simulink model "wlan_phy_rx_pmd"  (Simulink 7.8)
settings: Solver=VariableStepDiscrete  SolverMode=SingleTasking  StartTime=0.0  StopTime=rx_sim.sim_time  RelTol=1e-3
PostLoadFcn:
  | wlan_phy_rx_init
InitFcn:
  | wlan_phy_rx_init
StartFcn:
  | wlan_phy_rx_init
values from neighbouring files (symbols this model uses):
  barker_seq20 = 0.95 * circshift(barker_seq20, [0 4]) ./ max(abs(barker_seq20))    [wlan_phy_rx_init.m]
  bit_scrambler_lfsr_bytes = sum(bit_scrambler_lfsr_bytes .* repmat(2.^[0:7], 127, 1),2)    [wlan_phy_rx_init.m]
  longCorr_coef_i = [3*real(longCorr_coef)]    [wlan_phy_rx_init.m]
  longCorr_coef_q = [3*imag(longCorr_coef)]    [wlan_phy_rx_init.m]
  MAX_CP_LEN = 32    [wlan_phy_rx_init.m]
  MAX_NCBPS = NCBPS_11n_64QAM    (= 312)    [wlan_phy_rx_init.m]
  MAX_NUM_BYTES = 2^16-1    (= 65535)    [wlan_phy_rx_init.m]
  MAX_NUM_SC = 64    [wlan_phy_rx_init.m]
  MAX_NUM_SYMS = 600    [wlan_phy_rx_init.m]
  NDBPS_11a_BPSK12 = 24    [wlan_phy_rx_init.m]
  preFFT_sampBuff_numSamps = 4*MAX_NUM_SC    (= 256)    [wlan_phy_rx_init.m]
  rx_sim = struct()    [wlan_phy_rx_init.m]
  scr_ind_rev = zeros(1,128)    [wlan_phy_rx_init.m]
undefined symbols (not defined in the file or in its folder): gcbh, mcs_rom_11ag, mcs_rom_11n, wlan_rx_deinterleave_rom_gen, xlBlockLoadCallback, xlGetNormalizedPeriod, xlipgetpartsetting
libraries: xbsIndex_r4 x4943, xbsEDKLib_r4 x1
(part 17 of 28)

== system wlan_phy_rx_pmd/Sync & Antenna Sel/LTS Correlation/Correlator/C5 ==
  x : Inport
  x_valid : Inport  Port=2
  AddSub{1,4..7,12..13} (7 alike) : xbsIndex_r4/AddSub  mode=Addition  use_carryin=off  use_carryout=off  en=off  latency=1  precision=Full  arith_type=Unsigned  n_bits=8  bin_pt=2  quantization=Truncate  overflow=Wrap  dbl_ovrd=off  use_behavioral_HDL=on  hw_selection=Fabric  pipelined=off  use_rpm=off  block_type=addsub
      AddSub1 settings: xl_area=[3 6 0 5 0 0 0]  block_version=VER_STRING_GOES_HERE
      AddSub12 settings: xl_area=[3 5 0 4 0 0 0]  block_version=VER_STRING_GOES_HERE
      AddSub13 settings: xl_area=[3 6 0 5 0 0 0]  block_version=VER_STRING_GOES_HERE
      AddSub4 settings: xl_area=[4 7 0 6 0 0 0]  block_version=VER_STRING_GOES_HERE
      AddSub5 settings: xl_area=[3 6 0 5 0 0 0]  block_version=VER_STRING_GOES_HERE
      AddSub6 settings: xl_area=[3 5 0 4 0 0 0]  block_version=VER_STRING_GOES_HERE
      AddSub7 settings: xl_area=[3 5 0 4 0 0 0]  block_version=VER_STRING_GOES_HERE
  C1 : Subsystem  mask{h=h, c_ind=0, N=N}
      mask prompts: h "Coefficients (all)"; c_ind "Sub-correlator Ind"; N "Coef/correlator"
      mask init:
      | coef2 = h((c_ind*N+1) : (c_ind + 1)*N);
  C2 : Subsystem  mask{h=h, c_ind=1, N=N}
      mask prompts: h "Coefficients (all)"; c_ind "Sub-correlator Ind"; N "Coef/correlator"
      mask init:
      | coef2 = h((c_ind*N+1) : (c_ind + 1)*N);
  C3 : Subsystem  mask{h=h, c_ind=2, N=N}
      mask prompts: h "Coefficients (all)"; c_ind "Sub-correlator Ind"; N "Coef/correlator"
      mask init:
      | coef2 = h((c_ind*N+1) : (c_ind + 1)*N);
  C4 : Subsystem  mask{h=h, c_ind=3, N=N}
      mask prompts: h "Coefficients (all)"; c_ind "Sub-correlator Ind"; N "Coef/correlator"
      mask init:
      | coef2 = h((c_ind*N+1) : (c_ind + 1)*N);
  C5 : Subsystem  mask{h=h, c_ind=4, N=N}
      mask prompts: h "Coefficients (all)"; c_ind "Sub-correlator Ind"; N "Coef/correlator"
      mask init:
      | coef2 = h((c_ind*N+1) : (c_ind + 1)*N);
  C6 : Subsystem  mask{h=h, c_ind=5, N=N}
      mask prompts: h "Coefficients (all)"; c_ind "Sub-correlator Ind"; N "Coef/correlator"
      mask init:
      | coef2 = h((c_ind*N+1) : (c_ind + 1)*N);
  C7 : Subsystem  mask{h=h, c_ind=6, N=N}
      mask prompts: h "Coefficients (all)"; c_ind "Sub-correlator Ind"; N "Coef/correlator"
      mask init:
      | coef2 = h((c_ind*N+1) : (c_ind + 1)*N);
  C8 : Subsystem  mask{h=h, c_ind=7, N=N}
      mask prompts: h "Coefficients (all)"; c_ind "Sub-correlator Ind"; N "Coef/correlator"
      mask init:
      | coef2 = h((c_ind*N+1) : (c_ind + 1)*N);
  Gateway Out{1..14} (14 alike) : xbsIndex_r4/Gateway Out  inherit_from_input=off  hdl_port=off  timing_constraint=None  locs_specified=off  LOCs={}  UseAsDAC=off  DACChannel='1'  block_type=gatewayout
      settings: infoedit="Gateway out block.  Converts Xilinx fixed point inputs into ouputs of type Simulink integer, double, or fixed point.<P><P>Hardware notes:  In hardware these blocks become top level output ports or are discarded, depending on how they are configured."  xl_area=[0 0 0 0 0 0 0]  block_version=VER_STRING_GOES_HERE
  y : Outport
  y_vout : Outport  Port=2
  x_dly : Outport  Port=3
  x_dly_vout : Outport  Port=4
  wires:
  C8/2(x_dly_vout) -> x_dly_vout/1
  C8/1(x_dly) -> x_dly/1
  Gateway Out14/1 -> ?
  Gateway Out13/1 -> ?
  Gateway Out12/1 -> ?
  Gateway Out11/1 -> ?
  Gateway Out10/1 -> ?
  Gateway Out9/1 -> ?
  Gateway Out1/1 -> ?
  Gateway Out8/1 -> ?
  Gateway Out6/1 -> ?
  Gateway Out7/1 -> ?
  C8/4(y_vout) -> y_vout/1
  AddSub4/1 -> Gateway Out14/1, y/1, Gateway Out5/1
  AddSub1/1 -> AddSub4/1
  AddSub13/1 -> AddSub4/2
  AddSub7/1 -> AddSub1/2
  AddSub6/1 -> AddSub1/1
  C7/3(y) -> Gateway Out12/1, AddSub7/1
  C8/3(y) -> Gateway Out13/1, AddSub7/2
  C5/3(y) -> Gateway Out10/1, AddSub6/1
  C6/3(y) -> Gateway Out11/1, AddSub6/2
  C7/2(x_dly_vout) -> C8/2(vin)
  C7/1(x_dly) -> C8/1(x)
  C6/2(x_dly_vout) -> C7/2(vin)
  C6/1(x_dly) -> C7/1(x)
  C5/2(x_dly_vout) -> C6/2(vin)
  C5/1(x_dly) -> C6/1(x)
  C4/3(y) -> Gateway Out9/1, AddSub12/2
  C4/2(x_dly_vout) -> C5/2(vin)
  C4/1(x_dly) -> C5/1(x)
  C3/3(y) -> Gateway Out8/1, AddSub12/1
  C3/2(x_dly_vout) -> C4/2(vin)
  C3/1(x_dly) -> C4/1(x)
  C1/3(y) -> Gateway Out7/1, Gateway Out2/1, AddSub5/1
  C2/3(y) -> Gateway Out1/1, AddSub5/2
  C2/2(x_dly_vout) -> C3/2(vin)
  C2/1(x_dly) -> C3/1(x)
  C1/2(x_dly_vout) -> C2/2(vin)
  C1/1(x_dly) -> C2/1(x)
  x_valid/1 -> Gateway Out4/1, C1/2(vin)
  ? -> ?
  Gateway Out2/1 -> ?
  Gateway Out5/1 -> ?
  Gateway Out3/1 -> ?
  Gateway Out4/1 -> ?
  AddSub5/1 -> AddSub13/1
  AddSub12/1 -> AddSub13/2
  x/1 -> Gateway Out6/1, Gateway Out3/1, C1/1(x)

== system wlan_phy_rx_pmd/Sync & Antenna Sel/LTS Correlation/Correlator/C6 ==
  x : Inport
  x_valid : Inport  Port=2
  AddSub{1,4..7,12..13} (7 alike) : xbsIndex_r4/AddSub  mode=Addition  use_carryin=off  use_carryout=off  en=off  latency=1  precision=Full  arith_type=Unsigned  n_bits=8  bin_pt=2  quantization=Truncate  overflow=Wrap  dbl_ovrd=off  use_behavioral_HDL=on  hw_selection=Fabric  pipelined=off  use_rpm=off  block_type=addsub
      AddSub1 settings: xl_area=[3 6 0 5 0 0 0]  block_version=VER_STRING_GOES_HERE
      AddSub12 settings: xl_area=[3 5 0 4 0 0 0]  block_version=VER_STRING_GOES_HERE
      AddSub13 settings: xl_area=[3 6 0 5 0 0 0]  block_version=VER_STRING_GOES_HERE
      AddSub4 settings: xl_area=[4 7 0 6 0 0 0]  block_version=VER_STRING_GOES_HERE
      AddSub5 settings: xl_area=[3 6 0 5 0 0 0]  block_version=VER_STRING_GOES_HERE
      AddSub6 settings: xl_area=[3 5 0 4 0 0 0]  block_version=VER_STRING_GOES_HERE
      AddSub7 settings: xl_area=[3 5 0 4 0 0 0]  block_version=VER_STRING_GOES_HERE
  C1 : Subsystem  mask{h=h, c_ind=0, N=N}
      mask prompts: h "Coefficients (all)"; c_ind "Sub-correlator Ind"; N "Coef/correlator"
      mask init:
      | coef2 = h((c_ind*N+1) : (c_ind + 1)*N);
  C2 : Subsystem  mask{h=h, c_ind=1, N=N}
      mask prompts: h "Coefficients (all)"; c_ind "Sub-correlator Ind"; N "Coef/correlator"
      mask init:
      | coef2 = h((c_ind*N+1) : (c_ind + 1)*N);
  C3 : Subsystem  mask{h=h, c_ind=2, N=N}
      mask prompts: h "Coefficients (all)"; c_ind "Sub-correlator Ind"; N "Coef/correlator"
      mask init:
      | coef2 = h((c_ind*N+1) : (c_ind + 1)*N);
  C4 : Subsystem  mask{h=h, c_ind=3, N=N}
      mask prompts: h "Coefficients (all)"; c_ind "Sub-correlator Ind"; N "Coef/correlator"
      mask init:
      | coef2 = h((c_ind*N+1) : (c_ind + 1)*N);
  C5 : Subsystem  mask{h=h, c_ind=4, N=N}
      mask prompts: h "Coefficients (all)"; c_ind "Sub-correlator Ind"; N "Coef/correlator"
      mask init:
      | coef2 = h((c_ind*N+1) : (c_ind + 1)*N);
  C6 : Subsystem  mask{h=h, c_ind=5, N=N}
      mask prompts: h "Coefficients (all)"; c_ind "Sub-correlator Ind"; N "Coef/correlator"
      mask init:
      | coef2 = h((c_ind*N+1) : (c_ind + 1)*N);
  C7 : Subsystem  mask{h=h, c_ind=6, N=N}
      mask prompts: h "Coefficients (all)"; c_ind "Sub-correlator Ind"; N "Coef/correlator"
      mask init:
      | coef2 = h((c_ind*N+1) : (c_ind + 1)*N);
  C8 : Subsystem  mask{h=h, c_ind=7, N=N}
      mask prompts: h "Coefficients (all)"; c_ind "Sub-correlator Ind"; N "Coef/correlator"
      mask init:
      | coef2 = h((c_ind*N+1) : (c_ind + 1)*N);
  Gateway Out{1..14} (14 alike) : xbsIndex_r4/Gateway Out  inherit_from_input=off  hdl_port=off  timing_constraint=None  locs_specified=off  LOCs={}  UseAsDAC=off  DACChannel='1'  block_type=gatewayout
      settings: infoedit="Gateway out block.  Converts Xilinx fixed point inputs into ouputs of type Simulink integer, double, or fixed point.<P><P>Hardware notes:  In hardware these blocks become top level output ports or are discarded, depending on how they are configured."  xl_area=[0 0 0 0 0 0 0]  block_version=VER_STRING_GOES_HERE
  y : Outport
  y_vout : Outport  Port=2
  x_dly : Outport  Port=3
  x_dly_vout : Outport  Port=4
  wires:
  x/1 -> C1/1(x), Gateway Out3/1, Gateway Out6/1
  AddSub12/1 -> AddSub13/2
  AddSub5/1 -> AddSub13/1
  Gateway Out4/1 -> ?
  Gateway Out3/1 -> ?
  Gateway Out5/1 -> ?
  Gateway Out2/1 -> ?
  ? -> ?
  x_valid/1 -> C1/2(vin), Gateway Out4/1
  C1/1(x_dly) -> C2/1(x)
  C1/2(x_dly_vout) -> C2/2(vin)
  C2/1(x_dly) -> C3/1(x)
  C2/2(x_dly_vout) -> C3/2(vin)
  C2/3(y) -> AddSub5/2, Gateway Out1/1
  C1/3(y) -> AddSub5/1, Gateway Out2/1, Gateway Out7/1
  C3/1(x_dly) -> C4/1(x)
  C3/2(x_dly_vout) -> C4/2(vin)
  C3/3(y) -> AddSub12/1, Gateway Out8/1
  C4/1(x_dly) -> C5/1(x)
  C4/2(x_dly_vout) -> C5/2(vin)
  C4/3(y) -> AddSub12/2, Gateway Out9/1
  C5/1(x_dly) -> C6/1(x)
  C5/2(x_dly_vout) -> C6/2(vin)
  C6/1(x_dly) -> C7/1(x)
  C6/2(x_dly_vout) -> C7/2(vin)
  C7/1(x_dly) -> C8/1(x)
  C7/2(x_dly_vout) -> C8/2(vin)
  C6/3(y) -> AddSub6/2, Gateway Out11/1
  C5/3(y) -> AddSub6/1, Gateway Out10/1
  C8/3(y) -> AddSub7/2, Gateway Out13/1
  C7/3(y) -> AddSub7/1, Gateway Out12/1
  AddSub6/1 -> AddSub1/1
  AddSub7/1 -> AddSub1/2
  AddSub13/1 -> AddSub4/2
  AddSub1/1 -> AddSub4/1
  AddSub4/1 -> Gateway Out5/1, y/1, Gateway Out14/1
  C8/4(y_vout) -> y_vout/1
  Gateway Out7/1 -> ?
  Gateway Out6/1 -> ?
  Gateway Out8/1 -> ?
  Gateway Out1/1 -> ?
  Gateway Out9/1 -> ?
  Gateway Out10/1 -> ?
  Gateway Out11/1 -> ?
  Gateway Out12/1 -> ?
  Gateway Out13/1 -> ?
  Gateway Out14/1 -> ?
  C8/1(x_dly) -> x_dly/1
  C8/2(x_dly_vout) -> x_dly_vout/1

== system wlan_phy_rx_pmd/Sync & Antenna Sel/LTS Correlation/Correlator/C7 ==
  x : Inport
  x_valid : Inport  Port=2
  AddSub{1,4..7,12..13} (7 alike) : xbsIndex_r4/AddSub  mode=Addition  use_carryin=off  use_carryout=off  en=off  latency=1  precision=Full  arith_type=Unsigned  n_bits=8  bin_pt=2  quantization=Truncate  overflow=Wrap  dbl_ovrd=off  use_behavioral_HDL=on  hw_selection=Fabric  pipelined=off  use_rpm=off  block_type=addsub
      AddSub1 settings: xl_area=[3 6 0 5 0 0 0]  block_version=VER_STRING_GOES_HERE
      AddSub12 settings: xl_area=[3 5 0 4 0 0 0]  block_version=VER_STRING_GOES_HERE
      AddSub13 settings: xl_area=[3 6 0 5 0 0 0]  block_version=VER_STRING_GOES_HERE
      AddSub4 settings: xl_area=[4 7 0 6 0 0 0]  block_version=VER_STRING_GOES_HERE
      AddSub5 settings: xl_area=[3 6 0 5 0 0 0]  block_version=VER_STRING_GOES_HERE
      AddSub6 settings: xl_area=[3 5 0 4 0 0 0]  block_version=VER_STRING_GOES_HERE
      AddSub7 settings: xl_area=[3 5 0 4 0 0 0]  block_version=VER_STRING_GOES_HERE
  C1 : Subsystem  mask{h=h, c_ind=0, N=N}
      mask prompts: h "Coefficients (all)"; c_ind "Sub-correlator Ind"; N "Coef/correlator"
      mask init:
      | coef2 = h((c_ind*N+1) : (c_ind + 1)*N);
  C2 : Subsystem  mask{h=h, c_ind=1, N=N}
      mask prompts: h "Coefficients (all)"; c_ind "Sub-correlator Ind"; N "Coef/correlator"
      mask init:
      | coef2 = h((c_ind*N+1) : (c_ind + 1)*N);
  C3 : Subsystem  mask{h=h, c_ind=2, N=N}
      mask prompts: h "Coefficients (all)"; c_ind "Sub-correlator Ind"; N "Coef/correlator"
      mask init:
      | coef2 = h((c_ind*N+1) : (c_ind + 1)*N);
  C4 : Subsystem  mask{h=h, c_ind=3, N=N}
      mask prompts: h "Coefficients (all)"; c_ind "Sub-correlator Ind"; N "Coef/correlator"
      mask init:
      | coef2 = h((c_ind*N+1) : (c_ind + 1)*N);
  C5 : Subsystem  mask{h=h, c_ind=4, N=N}
      mask prompts: h "Coefficients (all)"; c_ind "Sub-correlator Ind"; N "Coef/correlator"
      mask init:
      | coef2 = h((c_ind*N+1) : (c_ind + 1)*N);
  C6 : Subsystem  mask{h=h, c_ind=5, N=N}
      mask prompts: h "Coefficients (all)"; c_ind "Sub-correlator Ind"; N "Coef/correlator"
      mask init:
      | coef2 = h((c_ind*N+1) : (c_ind + 1)*N);
  C7 : Subsystem  mask{h=h, c_ind=6, N=N}
      mask prompts: h "Coefficients (all)"; c_ind "Sub-correlator Ind"; N "Coef/correlator"
      mask init:
      | coef2 = h((c_ind*N+1) : (c_ind + 1)*N);
  C8 : Subsystem  mask{h=h, c_ind=7, N=N}
      mask prompts: h "Coefficients (all)"; c_ind "Sub-correlator Ind"; N "Coef/correlator"
      mask init:
      | coef2 = h((c_ind*N+1) : (c_ind + 1)*N);
  Gateway Out{1..14} (14 alike) : xbsIndex_r4/Gateway Out  inherit_from_input=off  hdl_port=off  timing_constraint=None  locs_specified=off  LOCs={}  UseAsDAC=off  DACChannel='1'  block_type=gatewayout
      settings: infoedit="Gateway out block.  Converts Xilinx fixed point inputs into ouputs of type Simulink integer, double, or fixed point.<P><P>Hardware notes:  In hardware these blocks become top level output ports or are discarded, depending on how they are configured."  xl_area=[0 0 0 0 0 0 0]  block_version=VER_STRING_GOES_HERE
  y : Outport
  y_vout : Outport  Port=2
  x_dly : Outport  Port=3
  x_dly_vout : Outport  Port=4
  wires:
  x/1 -> C1/1(x), Gateway Out3/1, Gateway Out6/1
  AddSub12/1 -> AddSub13/2
  AddSub5/1 -> AddSub13/1
  Gateway Out4/1 -> ?
  Gateway Out3/1 -> ?
  Gateway Out5/1 -> ?
  Gateway Out2/1 -> ?
  ? -> ?
  x_valid/1 -> C1/2(vin), Gateway Out4/1
  C1/1(x_dly) -> C2/1(x)
  C1/2(x_dly_vout) -> C2/2(vin)
  C2/1(x_dly) -> C3/1(x)
  C2/2(x_dly_vout) -> C3/2(vin)
  C2/3(y) -> AddSub5/2, Gateway Out1/1
  C1/3(y) -> AddSub5/1, Gateway Out2/1, Gateway Out7/1
  C3/1(x_dly) -> C4/1(x)
  C3/2(x_dly_vout) -> C4/2(vin)
  C3/3(y) -> AddSub12/1, Gateway Out8/1
  C4/1(x_dly) -> C5/1(x)
  C4/2(x_dly_vout) -> C5/2(vin)
  C4/3(y) -> AddSub12/2, Gateway Out9/1
  C5/1(x_dly) -> C6/1(x)
  C5/2(x_dly_vout) -> C6/2(vin)
  C6/1(x_dly) -> C7/1(x)
  C6/2(x_dly_vout) -> C7/2(vin)
  C7/1(x_dly) -> C8/1(x)
  C7/2(x_dly_vout) -> C8/2(vin)
  C6/3(y) -> AddSub6/2, Gateway Out11/1
  C5/3(y) -> AddSub6/1, Gateway Out10/1
  C8/3(y) -> AddSub7/2, Gateway Out13/1
  C7/3(y) -> AddSub7/1, Gateway Out12/1
  AddSub6/1 -> AddSub1/1
  AddSub7/1 -> AddSub1/2
  AddSub13/1 -> AddSub4/2
  AddSub1/1 -> AddSub4/1
  AddSub4/1 -> Gateway Out5/1, y/1, Gateway Out14/1
  C8/4(y_vout) -> y_vout/1
  Gateway Out7/1 -> ?
  Gateway Out6/1 -> ?
  Gateway Out8/1 -> ?
  Gateway Out1/1 -> ?
  Gateway Out9/1 -> ?
  Gateway Out10/1 -> ?
  Gateway Out11/1 -> ?
  Gateway Out12/1 -> ?
  Gateway Out13/1 -> ?
  Gateway Out14/1 -> ?
  C8/1(x_dly) -> x_dly/1
  C8/2(x_dly_vout) -> x_dly_vout/1

== system wlan_phy_rx_pmd/Sync & Antenna Sel/LTS Correlation/Correlator/C8 ==
  x : Inport
  x_valid : Inport  Port=2
  AddSub{1,4..7,12..13} (7 alike) : xbsIndex_r4/AddSub  mode=Addition  use_carryin=off  use_carryout=off  en=off  latency=1  precision=Full  arith_type=Unsigned  n_bits=8  bin_pt=2  quantization=Truncate  overflow=Wrap  dbl_ovrd=off  use_behavioral_HDL=on  hw_selection=Fabric  pipelined=off  use_rpm=off  block_type=addsub
      AddSub1 settings: xl_area=[3 6 0 5 0 0 0]  block_version=VER_STRING_GOES_HERE
      AddSub12 settings: xl_area=[3 5 0 4 0 0 0]  block_version=VER_STRING_GOES_HERE
      AddSub13 settings: xl_area=[3 6 0 5 0 0 0]  block_version=VER_STRING_GOES_HERE
      AddSub4 settings: xl_area=[4 7 0 6 0 0 0]  block_version=VER_STRING_GOES_HERE
      AddSub5 settings: xl_area=[3 6 0 5 0 0 0]  block_version=VER_STRING_GOES_HERE
      AddSub6 settings: xl_area=[3 5 0 4 0 0 0]  block_version=VER_STRING_GOES_HERE
      AddSub7 settings: xl_area=[3 5 0 4 0 0 0]  block_version=VER_STRING_GOES_HERE
  C1 : Subsystem  mask{h=h, c_ind=0, N=N}
      mask prompts: h "Coefficients (all)"; c_ind "Sub-correlator Ind"; N "Coef/correlator"
      mask init:
      | coef2 = h((c_ind*N+1) : (c_ind + 1)*N);
  C2 : Subsystem  mask{h=h, c_ind=1, N=N}
      mask prompts: h "Coefficients (all)"; c_ind "Sub-correlator Ind"; N "Coef/correlator"
      mask init:
      | coef2 = h((c_ind*N+1) : (c_ind + 1)*N);
  C3 : Subsystem  mask{h=h, c_ind=2, N=N}
      mask prompts: h "Coefficients (all)"; c_ind "Sub-correlator Ind"; N "Coef/correlator"
      mask init:
      | coef2 = h((c_ind*N+1) : (c_ind + 1)*N);
  C4 : Subsystem  mask{h=h, c_ind=3, N=N}
      mask prompts: h "Coefficients (all)"; c_ind "Sub-correlator Ind"; N "Coef/correlator"
      mask init:
      | coef2 = h((c_ind*N+1) : (c_ind + 1)*N);
  C5 : Subsystem  mask{h=h, c_ind=4, N=N}
      mask prompts: h "Coefficients (all)"; c_ind "Sub-correlator Ind"; N "Coef/correlator"
      mask init:
      | coef2 = h((c_ind*N+1) : (c_ind + 1)*N);
  C6 : Subsystem  mask{h=h, c_ind=5, N=N}
      mask prompts: h "Coefficients (all)"; c_ind "Sub-correlator Ind"; N "Coef/correlator"
      mask init:
      | coef2 = h((c_ind*N+1) : (c_ind + 1)*N);
  C7 : Subsystem  mask{h=h, c_ind=6, N=N}
      mask prompts: h "Coefficients (all)"; c_ind "Sub-correlator Ind"; N "Coef/correlator"
      mask init:
      | coef2 = h((c_ind*N+1) : (c_ind + 1)*N);
  C8 : Subsystem  mask{h=h, c_ind=7, N=N}
      mask prompts: h "Coefficients (all)"; c_ind "Sub-correlator Ind"; N "Coef/correlator"
      mask init:
      | coef2 = h((c_ind*N+1) : (c_ind + 1)*N);
  Gateway Out{1..14} (14 alike) : xbsIndex_r4/Gateway Out  inherit_from_input=off  hdl_port=off  timing_constraint=None  locs_specified=off  LOCs={}  UseAsDAC=off  DACChannel='1'  block_type=gatewayout
      settings: infoedit="Gateway out block.  Converts Xilinx fixed point inputs into ouputs of type Simulink integer, double, or fixed point.<P><P>Hardware notes:  In hardware these blocks become top level output ports or are discarded, depending on how they are configured."  xl_area=[0 0 0 0 0 0 0]  block_version=VER_STRING_GOES_HERE
  y : Outport
  y_vout : Outport  Port=2
  x_dly : Outport  Port=3
  x_dly_vout : Outport  Port=4
  wires:
  C8/2(x_dly_vout) -> x_dly_vout/1
  C8/1(x_dly) -> x_dly/1
  Gateway Out14/1 -> ?
  Gateway Out13/1 -> ?
  Gateway Out12/1 -> ?
  Gateway Out11/1 -> ?
  Gateway Out10/1 -> ?
  Gateway Out9/1 -> ?
  Gateway Out1/1 -> ?
  Gateway Out8/1 -> ?
  Gateway Out6/1 -> ?
  Gateway Out7/1 -> ?
  C8/4(y_vout) -> y_vout/1
  AddSub4/1 -> Gateway Out14/1, y/1, Gateway Out5/1
  AddSub1/1 -> AddSub4/1
  AddSub13/1 -> AddSub4/2
  AddSub7/1 -> AddSub1/2
  AddSub6/1 -> AddSub1/1
  C7/3(y) -> Gateway Out12/1, AddSub7/1
  C8/3(y) -> Gateway Out13/1, AddSub7/2
  C5/3(y) -> Gateway Out10/1, AddSub6/1
  C6/3(y) -> Gateway Out11/1, AddSub6/2
  C7/2(x_dly_vout) -> C8/2(vin)
  C7/1(x_dly) -> C8/1(x)
  C6/2(x_dly_vout) -> C7/2(vin)
  C6/1(x_dly) -> C7/1(x)
  C5/2(x_dly_vout) -> C6/2(vin)
  C5/1(x_dly) -> C6/1(x)
  C4/3(y) -> Gateway Out9/1, AddSub12/2
  C4/2(x_dly_vout) -> C5/2(vin)
  C4/1(x_dly) -> C5/1(x)
  C3/3(y) -> Gateway Out8/1, AddSub12/1
  C3/2(x_dly_vout) -> C4/2(vin)
  C3/1(x_dly) -> C4/1(x)
  C1/3(y) -> Gateway Out7/1, Gateway Out2/1, AddSub5/1
  C2/3(y) -> Gateway Out1/1, AddSub5/2
  C2/2(x_dly_vout) -> C3/2(vin)
  C2/1(x_dly) -> C3/1(x)
  C1/2(x_dly_vout) -> C2/2(vin)
  C1/1(x_dly) -> C2/1(x)
  x_valid/1 -> Gateway Out4/1, C1/2(vin)
  ? -> ?
  Gateway Out2/1 -> ?
  Gateway Out5/1 -> ?
  Gateway Out3/1 -> ?
  Gateway Out4/1 -> ?
  AddSub5/1 -> AddSub13/1
  AddSub12/1 -> AddSub13/2
  x/1 -> Gateway Out6/1, Gateway Out3/1, C1/1(x)

== system wlan_phy_rx_pmd/Sync & Antenna Sel/LTS Correlation/Correlator/Combine ==
  Re*Re : Inport
  Im*Im : Inport  Port=2
  Re*Im : Inport  Port=3
  Im*Re : Inport  Port=4
  AddSub : xbsIndex_r4/AddSub  mode=Subtraction  use_carryin=off  use_carryout=off  en=off  latency=0  precision=Full  arith_type=Unsigned  n_bits=8  bin_pt=2  quantization=Truncate  overflow=Wrap  dbl_ovrd=off  use_behavioral_HDL=on  hw_selection=Fabric  pipelined=off  use_rpm=off  block_type=addsub
      settings: xl_area=[5 0 0 8 0 0 0]  block_version=VER_STRING_GOES_HERE
  AddSub1 : xbsIndex_r4/AddSub  mode=Addition  use_carryin=off  use_carryout=off  en=off  latency=0  precision=Full  arith_type=Unsigned  n_bits=8  bin_pt=2  quantization=Truncate  overflow=Wrap  dbl_ovrd=off  use_behavioral_HDL=on  hw_selection=Fabric  pipelined=off  use_rpm=off  block_type=addsub
      settings: xl_area=[5 0 0 8 0 0 0]  block_version=VER_STRING_GOES_HERE
  AddSub2 : xbsIndex_r4/AddSub  mode=Addition  use_carryin=off  use_carryout=off  en=off  latency=1  precision=User Defined  arith_type=Unsigned  n_bits=32  bin_pt=0  quantization=Truncate  overflow=Flag as error  dbl_ovrd=off  use_behavioral_HDL=on  hw_selection=Fabric  pipelined=off  use_rpm=off  block_type=addsub
      settings: xl_area=[10 0 0 18 0 0 0]  block_version=VER_STRING_GOES_HERE
  Bus Creator : BusCreator  Inputs=2
  Gateway Out{1..7} (7 alike) : xbsIndex_r4/Gateway Out  inherit_from_input=off  hdl_port=off  timing_constraint=None  locs_specified=off  LOCs={}  UseAsDAC=off  DACChannel='1'  block_type=gatewayout
  LTS Sum : Scope  NumInputPorts=8
      settings: TimeRange=5000  YMin=-50~-100~-40~-20~0~0~0~0  YMax=100~50~40~30~25000~2500~25000~25000  SaveName=ScopeData39
  Mult : xbsIndex_r4/Mult  precision=User Defined  arith_type=Unsigned  n_bits=32  bin_pt=0  quantization=Truncate  overflow=Flag as error  en=off  latency=1  dbl_ovrd=off  use_behavioral_HDL=off  opt=Speed  use_embedded=on  optimum_pipeline=off  pipeline=on  use_rpm=off  placement_style=Rectangular shape  block_type=mult
      settings: xl_area=[60 18 0 106 0 0 0]  block_version=VER_STRING_GOES_HERE
  Mult1 : xbsIndex_r4/Mult  precision=User Defined  arith_type=Unsigned  n_bits=32  bin_pt=0  quantization=Truncate  overflow=Flag as error  en=off  latency=1  dbl_ovrd=off  use_behavioral_HDL=off  opt=Speed  use_embedded=on  optimum_pipeline=off  pipeline=on  use_rpm=off  placement_style=Rectangular shape  block_type=mult
      settings: xl_area=[60 18 0 106 0 0 0]  block_version=VER_STRING_GOES_HERE
  R : Outport
  wires:
  AddSub/1 -> Mult/1, Mult/2
  AddSub1/1 -> Mult1/1, Mult1/2
  Mult/1 -> AddSub2/1, Gateway Out5/1
  Mult1/1 -> AddSub2/2, Gateway Out6/1
  Re*Re/1 -> AddSub/1, Gateway Out4/1
  Im*Im/1 -> AddSub/2, Gateway Out1/1
  AddSub2/1 -> R/1, Gateway Out7/1
  Re*Im/1 -> AddSub1/1, Gateway Out2/1
  Im*Re/1 -> AddSub1/2, Gateway Out3/1
  Gateway Out4/1 -> LTS Sum/1
  Gateway Out1/1 -> LTS Sum/2
  Gateway Out2/1 -> LTS Sum/3
  Gateway Out3/1 -> LTS Sum/4
  Gateway Out5/1 -> LTS Sum/5
  Gateway Out6/1 -> LTS Sum/6
  Gateway Out7/1 -> LTS Sum/8, Bus Creator/1, Bus Creator/2
  Bus Creator/1 -> LTS Sum/7

== system wlan_phy_rx_pmd/Sync & Antenna Sel/LTS Correlation/Correlator/FSM ==
  note: The correlator archiecture divides the 64-point dot product
      | into 8 parallel 8-point proudct/sum operations. For every sample
      | each sub block computes an 8-point dot product, the results of which
      | are then summed. For a sample rate = sys_clk/8 the correaltor runs
      | non-stop. For slower sample rates the correlator runs for 8 cycles
      | after each input sample, then pauses until the next sample is valid.
  IQ_Valid : Inport
  Coef Addr Gen : xbsIndex_r4/Counter  cnt_type=Free Running  cnt_to=N-1  operation=Up  start_count=0  cnt_by_val=1  arith_type=Unsigned  n_bits=ceil(log2(N))  bin_pt=0  load_pin=off  rst=off  en=on  explicit_period=off  period=1  dbl_ovrd=off  use_behavioral_HDL=off  implementation=Fabric  use_rpm=on  block_type=counter
      settings: xl_area=[3 3 0 4 0 0 0]  block_version=VER_STRING_GOES_HERE
  Constant : xbsIndex_r4/Constant  const=N-2  gui_display_data_type=Fixed-point  arith_type=Unsigned  n_bits=ceil(log2(N))  bin_pt=0  preci_type=Single  exp_width=8  frac_width=24  explicit_period=off  period=1  equ=P=C  opselect=C  inp2=PCIN>>17  opr=+  inp1=P  carry=CIN  dbl_ovrd=off  block_type=constant
  Data Addr Gen : xbsIndex_r4/Counter  cnt_type=Free Running  cnt_to=N-1  operation=Up  start_count=7  cnt_by_val=1  arith_type=Unsigned  n_bits=ceil(log2(N))  bin_pt=0  load_pin=off  rst=off  en=on  explicit_period=off  period=1  dbl_ovrd=off  use_behavioral_HDL=off  implementation=Fabric  use_rpm=on  block_type=counter
      settings: xl_area=[3 3 0 4 0 0 0]  block_version=VER_STRING_GOES_HERE
  Delay1 : xbsIndex_r4/Delay  rst=off  infoeditControl=Selection of Reset will increase slice count due to use of real FFs and instead of SRLs  en=off  latency=N  dbl_ovrd=off  reg_retiming=off  block_type=delay
  Gateway Out{,1..4} (5 alike) : xbsIndex_r4/Gateway Out  inherit_from_input=off  hdl_port=off  timing_constraint=None  locs_specified=off  LOCs={}  UseAsDAC=off  DACChannel='1'  block_type=gatewayout
      settings: infoedit="Gateway out block.  Converts Xilinx fixed point inputs into ouputs of type Simulink integer, double, or fixed point.<P><P>Hardware notes:  In hardware these blocks become top level output ports or are discarded, depending on how they are configured."  xl_area=[0 0 0 0 0 0 0]  block_version=VER_STRING_GOES_HERE
  Inverter : xbsIndex_r4/Inverter  en=off  latency=0  dbl_ovrd=off  block_type=inv
  Logical : xbsIndex_r4/Logical  logical_function=OR  inputs=2  en=off  latency=0  precision=Full  arith_type=Unsigned  n_bits=16  bin_pt=0  align_bp=on  dbl_ovrd=off  block_type=logical
  Logical1 : xbsIndex_r4/Logical  logical_function=AND  inputs=2  en=off  latency=0  precision=Full  arith_type=Unsigned  n_bits=16  bin_pt=0  align_bp=on  dbl_ovrd=off  block_type=logical
  Long Corr Scope : Scope  NumInputPorts=5
      settings: YMin=-1~-1~-35~-5~-5  YMax=1~1~25~5~5  SaveName=ScopeData4
  Relational : xbsIndex_r4/Relational  mode=a=b  en=off  latency=0  dbl_ovrd=off  block_type=relational
  S-R Latch : Subsystem
  Data Addr : Outport
  Coef Addr : Outport  Port=2
  load : Outport  Port=3
  wires:
  Gateway Out3/1 -> Long Corr Scope/3  "Corr Load"
  IQ_Valid/1 -> Delay1/1, S-R Latch/1(S), Inverter/1, Gateway Out2/1, Logical/1
  Gateway Out2/1 -> Long Corr Scope/4  "IQ  Valid"
  Delay1/1 -> load/1, Gateway Out3/1
  S-R Latch/1(Q) -> Logical/2
  Logical/1 -> Data Addr Gen/1, Coef Addr Gen/1, Gateway Out4/1
  Constant/1 -> Relational/2
  Relational/1 -> Logical1/2
  Logical1/1 -> S-R Latch/2(R)
  Inverter/1 -> Logical1/1
  Gateway Out4/1 -> Long Corr Scope/5  "Counters en"
  Data Addr Gen/1 -> Relational/1, Data Addr/1, Gateway Out/1
  Coef Addr Gen/1 -> Coef Addr/1, Gateway Out1/1
  Gateway Out/1 -> Long Corr Scope/1  "Data Addr"
  Gateway Out1/1 -> Long Corr Scope/2  "Coef Addr"

== system wlan_phy_rx_pmd/Sync & Antenna Sel/LTS Correlation/LTS Corr Timeout/S-R Latch ==
  S : Inport
  R : Inport  Port=2
  Constant1 : xbsIndex_r4/Constant  const=1  gui_display_data_type=Fixed-point  arith_type=Boolean  n_bits=1  bin_pt=0  preci_type=Single  exp_width=8  frac_width=24  explicit_period=off  period=1  equ=P=C  opselect=C  inp2=PCIN>>17  opr=+  inp1=P  carry=CIN  dbl_ovrd=off  block_type=constant
      settings: block_version=VER_STRING_GOES_HERE
  Convert1 : xbsIndex_r4/Convert  gui_display_data_type=Boolean  arith_type=Boolean  n_bits=1  bin_pt=0  float_type=Single  exp_bits=8  fraction_bits=24  quantization=Truncate  overflow=Wrap  en=off  latency=0  dbl_ovrd=off  pipeline=off  block_type=convert
  Convert2 : xbsIndex_r4/Convert  gui_display_data_type=Boolean  arith_type=Boolean  n_bits=1  bin_pt=0  float_type=Single  exp_bits=8  fraction_bits=24  quantization=Truncate  overflow=Wrap  en=off  latency=0  dbl_ovrd=off  pipeline=off  block_type=convert
  Register2 : xbsIndex_r4/Register  init=0  rst=on  en=on  dbl_ovrd=off  block_type=register
      settings: xl_area=[1 1 0 0 0 0 0]  block_version=VER_STRING_GOES_HERE
  Q : Outport
  wires:
  S/1 -> Convert2/1
  Register2/1 -> Q/1
  R/1 -> Convert1/1
  Constant1/1 -> Register2/1
  Convert1/1 -> Register2/2
  Convert2/1 -> Register2/3

== system wlan_phy_rx_pmd/Sync & Antenna Sel/LTS Correlation/LTS Corr Timeout/S-R Latch1 ==
  S : Inport
  R : Inport  Port=2
  Constant1 : xbsIndex_r4/Constant  const=1  gui_display_data_type=Fixed-point  arith_type=Boolean  n_bits=1  bin_pt=0  preci_type=Single  exp_width=8  frac_width=24  explicit_period=off  period=1  equ=P=C  opselect=C  inp2=PCIN>>17  opr=+  inp1=P  carry=CIN  dbl_ovrd=off  block_type=constant
      settings: block_version=VER_STRING_GOES_HERE
  Convert1 : xbsIndex_r4/Convert  gui_display_data_type=Boolean  arith_type=Boolean  n_bits=1  bin_pt=0  float_type=Single  exp_bits=8  fraction_bits=24  quantization=Truncate  overflow=Wrap  en=off  latency=0  dbl_ovrd=off  pipeline=off  block_type=convert
  Convert2 : xbsIndex_r4/Convert  gui_display_data_type=Boolean  arith_type=Boolean  n_bits=1  bin_pt=0  float_type=Single  exp_bits=8  fraction_bits=24  quantization=Truncate  overflow=Wrap  en=off  latency=0  dbl_ovrd=off  pipeline=off  block_type=convert
  Register2 : xbsIndex_r4/Register  init=0  rst=on  en=on  dbl_ovrd=off  block_type=register
      settings: xl_area=[1 1 0 0 0 0 0]  block_version=VER_STRING_GOES_HERE
  Q : Outport
  wires:
  Convert2/1 -> Register2/3
  Convert1/1 -> Register2/2
  Constant1/1 -> Register2/1
  R/1 -> Convert1/1
  Register2/1 -> Q/1
  S/1 -> Convert2/1

== system wlan_phy_rx_pmd/Sync & Antenna Sel/LTS Correlation/Threshold Select/posedge ==
  D : Inport
  Delay : xbsIndex_r4/Delay  rst=off  infoeditControl=Selection of Reset will increase slice count due to use of real FFs and instead of SRLs  en=off  latency=1  dbl_ovrd=off  reg_retiming=off  block_type=delay
      settings: infoedit=Hardware notes: A delay line is a chain, each link of which is an SRL16 followed by a flip-flop. If register retiming is enabled, the delay line is a chain of flip-flops.  xl_area=[1 1 0 0 0 0 0]  block_version=VER_STRING_GOES_HERE
  Inverter : xbsIndex_r4/Inverter  en=off  latency=0  dbl_ovrd=off  block_type=inv
      settings: xl_area=[0 0 0 0 0 0 0]  block_version=VER_STRING_GOES_HERE
  Logical1 : xbsIndex_r4/Logical  logical_function=AND  inputs=2  en=off  latency=0  precision=Full  arith_type=Unsigned  n_bits=8  bin_pt=2  align_bp=on  dbl_ovrd=off  block_type=logical
      settings: xl_area=[1 0 0 1 0 0 0]  block_version=VER_STRING_GOES_HERE
  Q : Outport
  wires:
  Delay/1 -> Inverter/1
  Logical1/1 -> Q/1
  D/1 -> Delay/1, Logical1/2
  Inverter/1 -> Logical1/1

== system wlan_phy_rx_pmd/Sync & Antenna Sel/PHY CCA/Rate-Length BUSY/Bits ==
  Sym Inc : Inport
  Rst : Inport  Port=2
  Accumulator : xbsIndex_r4/Accumulator  operation=Add  n_bits=ceil(log2(MAX_NUM_BYTES*8))  overflow=Wrap  scale=1  rst=on  infoeditControl=reset for floating point data type must be asserted for a minimum of 2 cycles  hasbypass=on  en=on  latency=0  dbl_ovrd=off  msb_inp=100  msb=100  lsb=-100  use_behavioral_HDL=on  implementation=Fabric  block_type=accum
  Gateway Out{1..6,8} (7 alike) : xbsIndex_r4/Gateway Out  inherit_from_input=off  hdl_port=off  timing_constraint=None  locs_specified=off  LOCs={}  UseAsDAC=off  DACChannel='1'  block_type=gatewayout
  Logical4 : xbsIndex_r4/Logical  logical_function=AND  inputs=2  en=off  latency=0  precision=Full  arith_type=Unsigned  n_bits=16  bin_pt=0  align_bp=on  dbl_ovrd=off  block_type=logical
  Logical5 : xbsIndex_r4/Logical  logical_function=OR  inputs=2  en=off  latency=0  precision=Full  arith_type=Unsigned  n_bits=16  bin_pt=0  align_bp=on  dbl_ovrd=off  block_type=logical
  PHY Rx Busy - Bits : Scope  NumInputPorts=7
      settings: TimeRange=20000  YMin=0~20~0~0~0~0~0  YMax=120~50~1~1000~1~1~1  SaveName=ScopeData23
  Posedge : Subsystem
  Register : xbsIndex_r4/Register  init=24  rst=on  en=on  dbl_ovrd=off  block_type=register
  Register1 : xbsIndex_r4/Register  init=9  rst=on  en=on  dbl_ovrd=off  block_type=register
  Relational2 : xbsIndex_r4/Relational  mode=a<b  en=off  latency=0  dbl_ovrd=off  block_type=relational
  S-R Latch3 : Subsystem
  Target Num Bits : Subsystem
  Done : Outport
  wires:
  [OFDM_RX_DATA_N_DBPS_1] -> Register/1
  S-R Latch3/1(Q) -> Logical4/1
  Register/1 -> Accumulator/1, Gateway Out2/1
  Logical4/1 -> Accumulator/3
  Accumulator/1 -> Relational2/2, Gateway Out3/1
  Register1/1 -> Gateway Out1/1, Target Num Bits/1(SIG.LENGTH)
  Target Num Bits/1(Total Bits) -> Relational2/1
  Logical5/1 -> Accumulator/2, S-R Latch3/2(R), Register/2, Register1/2
  Rst/1 -> Logical5/1, Gateway Out8/1
  Relational2/1 -> Done/1, Logical5/2, Gateway Out4/1
  Gateway Out8/1 -> PHY Rx Busy - Bits/7  "Reset"
  Gateway Out1/1 -> PHY Rx Busy - Bits/1  "Length (Bytes)"
  Gateway Out2/1 -> PHY Rx Busy - Bits/2  "N_DBPS"
  Gateway Out3/1 -> PHY Rx Busy - Bits/4  "Bit Accum"
  Gateway Out4/1 -> PHY Rx Busy - Bits/5  "Done"
  Gateway Out5/1 -> PHY Rx Busy - Bits/6  "Accum Inc En"
  [OFDM_RX_SIGNAL_LENGTH] -> Register1/1
  Posedge/1(Q) -> Register/3, S-R Latch3/1(S), Register1/3, Gateway Out6/1
  Sym Inc/1 -> Logical4/2, Gateway Out5/1
  Gateway Out6/1 -> PHY Rx Busy - Bits/3  "SIGNAL Valid"
  [OFDM_RX_SIGNAL_VALID] -> Posedge/1(D)

== system wlan_phy_rx_pmd/Sync & Antenna Sel/PHY CCA/Rate-Length BUSY/Extension ==
  note: HT waveforms have extra mid-pkt training symbols:
      | 1 HT-STF (4 usec)
      | N_HT-LTF (4 usec):
      | N_SS = [1,2,3] -> N_HT-LTF = [1,2,4]
      |
      | Durations of HT-SIG1/2 are handled in the upstream "Bits"
  Bits Done : Inport
  IQ Valid : Inport  Port=2
  Rst : Inport  Port=3
  AddSub : xbsIndex_r4/AddSub  mode=Addition  use_carryin=off  use_carryout=off  en=off  latency=1  precision=Full  arith_type=Unsigned  n_bits=16  bin_pt=14  quantization=Truncate  overflow=Wrap  dbl_ovrd=off  use_behavioral_HDL=off  hw_selection=Fabric  pipelined=off  block_type=addsub
  Concat : xbsIndex_r4/Concat  num_inputs=2  dbl_ovrd=off  block_type=concat
  Constant1 : xbsIndex_r4/Constant  const=80+1*80  gui_display_data_type=Fixed-point  arith_type=Unsigned  n_bits=8  bin_pt=0  preci_type=Single  exp_width=8  frac_width=24  explicit_period=off  period=1  equ=P=C  opselect=C  inp2=PCIN>>17  opr=+  inp1=P  carry=CIN  dbl_ovrd=off  block_type=constant
      settings: block_version=VER_STRING_GOES_HERE
  Constant2 : xbsIndex_r4/Constant  const=0  gui_display_data_type=Fixed-point  arith_type=Unsigned  n_bits=1  bin_pt=0  preci_type=Single  exp_width=8  frac_width=24  explicit_period=off  period=1  equ=P=C  opselect=C  inp2=PCIN>>17  opr=+  inp1=P  carry=CIN  dbl_ovrd=off  block_type=constant
      settings: block_version=VER_STRING_GOES_HERE
  Constant3 : xbsIndex_r4/Constant  const=80+2*80  gui_display_data_type=Fixed-point  arith_type=Unsigned  n_bits=8  bin_pt=0  preci_type=Single  exp_width=8  frac_width=24  explicit_period=off  period=1  equ=P=C  opselect=C  inp2=PCIN>>17  opr=+  inp1=P  carry=CIN  dbl_ovrd=off  block_type=constant
      settings: block_version=VER_STRING_GOES_HERE
  Constant4 : xbsIndex_r4/Constant  const=80+3*80  gui_display_data_type=Fixed-point  arith_type=Unsigned  n_bits=9  bin_pt=0  preci_type=Single  exp_width=8  frac_width=24  explicit_period=off  period=1  equ=P=C  opselect=C  inp2=PCIN>>17  opr=+  inp1=P  carry=CIN  dbl_ovrd=off  block_type=constant
      settings: block_version=VER_STRING_GOES_HERE
  Constant5 : xbsIndex_r4/Constant  const=0  gui_display_data_type=Fixed-point  arith_type=Unsigned  n_bits=2  bin_pt=0  preci_type=Single  exp_width=8  frac_width=24  explicit_period=on  period=1  equ=P=C  opselect=C  inp2=PCIN>>17  opr=+  inp1=P  carry=CIN  dbl_ovrd=off  block_type=constant
      settings: block_version=VER_STRING_GOES_HERE
  Counter1 : xbsIndex_r4/Counter  cnt_type=Free Running  cnt_to=Inf  operation=Up  start_count=0  cnt_by_val=1  arith_type=Unsigned  n_bits=11  bin_pt=0  load_pin=off  rst=on  en=on  explicit_period=off  period=1  dbl_ovrd=off  use_behavioral_HDL=off  implementation=Fabric  block_type=counter
  Display : Display
  Gateway Out{1..6} (6 alike) : xbsIndex_r4/Gateway Out  inherit_from_input=off  hdl_port=off  timing_constraint=None  locs_specified=off  LOCs={}  UseAsDAC=off  DACChannel='1'  block_type=gatewayout
  Logical1 : xbsIndex_r4/Logical  logical_function=AND  inputs=2  en=off  latency=0  precision=Full  arith_type=Unsigned  n_bits=16  bin_pt=0  align_bp=on  dbl_ovrd=off  block_type=logical
  Logical2 : xbsIndex_r4/Logical  logical_function=OR  inputs=2  en=off  latency=0  precision=Full  arith_type=Unsigned  n_bits=16  bin_pt=0  align_bp=on  dbl_ovrd=off  block_type=logical
  Logical3 : xbsIndex_r4/Logical  logical_function=AND  inputs=2  en=off  latency=0  precision=Full  arith_type=Unsigned  n_bits=16  bin_pt=0  align_bp=on  dbl_ovrd=off  block_type=logical
  Logical4 : xbsIndex_r4/Logical  logical_function=AND  inputs=2  en=off  latency=0  precision=Full  arith_type=Unsigned  n_bits=16  bin_pt=0  align_bp=on  dbl_ovrd=off  block_type=logical
  Mux : xbsIndex_r4/Mux  inputs=4  en=off  latency=0  precision=Full  arith_type=Unsigned  n_bits=16  bin_pt=14  quantization=Truncate  overflow=Wrap  dbl_ovrd=off  block_type=mux
  PHY Rx Busy - Extension : Scope  NumInputPorts=7
      settings: TimeRange=10000  YMin=0~-100~-100~-70~0~0~-0.25  YMax=80~75~75~80~1~60000~0.3  SaveName=ScopeData33
  Register2 : xbsIndex_r4/Register  init=0  rst=on  en=on  dbl_ovrd=off  block_type=register
  Relational3 : xbsIndex_r4/Relational  mode=a>=b  en=off  latency=0  dbl_ovrd=off  block_type=relational
  S-R Latch1 : Subsystem
  Pkt Done : Outport
  wires:
  Relational3/1 -> Logical2/1, Logical3/2
  Counter1/1 -> Relational3/1, Gateway Out5/1
  Logical2/1 -> Counter1/1, S-R Latch1/2(R)
  Rst/1 -> Logical2/2, Register2/2
  Bits Done/1 -> Gateway Out4/1, S-R Latch1/1(S)
  S-R Latch1/1(Q) -> Logical1/1, Logical3/1
  IQ Valid/1 -> Logical1/2
  Logical3/1 -> Pkt Done/1, Gateway Out3/1
  Gateway Out4/1 -> PHY Rx Busy - Extension/1
  Gateway Out1/1 -> PHY Rx Busy - Extension/2
  Gateway Out2/1 -> PHY Rx Busy - Extension/3
  Gateway Out3/1 -> PHY Rx Busy - Extension/4
  Concat/1 -> Logical4/1
  [OFDM_RX_DATA_N_SS] -> Logical4/2
  [RX_PHY_MODE_11N] -> Concat/1, Concat/2
  Constant2/1 -> Mux/2
  Constant1/1 -> Mux/3
  Constant3/1 -> Mux/4
  Constant4/1 -> Mux/5
  [regRx_CCA_PostRx_Extension] -> AddSub/1, Gateway Out6/1
  Mux/1 -> AddSub/2
  AddSub/1 -> Relational3/2, Gateway Out2/1
  Logical1/1 -> Counter1/2, Gateway Out1/1
  Gateway Out5/1 -> PHY Rx Busy - Extension/5
  Register2/1 -> ?
  Logical4/1 -> Register2/1
  [OFDM_RX_PARAMS_READY] -> Register2/3
  Gateway Out6/1 -> Display/1
  Constant5/1 -> Mux/1

== system wlan_phy_rx_pmd/Sync & Antenna Sel/PHY CCA/Rate-Length BUSY/OFDM Syms ==
  Start : Inport
  IQ valid : Inport  Port=2
  Rst : Inport  Port=3
  AddSub : xbsIndex_r4/AddSub  mode=Addition  use_carryin=off  use_carryout=off  en=off  latency=0  precision=Full  arith_type=Unsigned  n_bits=16  bin_pt=14  quantization=Truncate  overflow=Wrap  dbl_ovrd=off  use_behavioral_HDL=off  hw_selection=Fabric  pipelined=off  block_type=addsub
  Counter : xbsIndex_r4/Counter  cnt_type=Free Running  cnt_to=Inf  operation=Up  start_count=1  cnt_by_val=1  arith_type=Unsigned  n_bits=ceil(log2(MAX_CP_LEN)) + ceil(log2(MAX_NUM_SC))  bin_pt=0  load_pin=off  rst=on  en=on  explicit_period=off  period=1  dbl_ovrd=off  use_behavioral_HDL=off  implementation=Fabric  block_type=counter
  Display : Display
  Gateway Out9 : xbsIndex_r4/Gateway Out  inherit_from_input=off  hdl_port=off  timing_constraint=None  locs_specified=off  LOCs={}  UseAsDAC=off  DACChannel='1'  block_type=gatewayout
  Inverter : xbsIndex_r4/Inverter  en=off  latency=0  dbl_ovrd=off  block_type=inv
      settings: xl_area=[0 0 0 0 0 0 0]  block_version=VER_STRING_GOES_HERE
  Logical1 : xbsIndex_r4/Logical  logical_function=AND  inputs=2  en=off  latency=0  precision=Full  arith_type=Unsigned  n_bits=16  bin_pt=0  align_bp=on  dbl_ovrd=off  block_type=logical
  Logical2 : xbsIndex_r4/Logical  logical_function=AND  inputs=2  en=off  latency=0  precision=Full  arith_type=Unsigned  n_bits=16  bin_pt=0  align_bp=on  dbl_ovrd=off  block_type=logical
  Logical3 : xbsIndex_r4/Logical  logical_function=OR  inputs=2  en=off  latency=0  precision=Full  arith_type=Unsigned  n_bits=16  bin_pt=0  align_bp=on  dbl_ovrd=off  block_type=logical
  Relational1 : xbsIndex_r4/Relational  mode=a<=b  en=off  latency=0  dbl_ovrd=off  block_type=relational
  S-R Latch1 : Subsystem
  Sym : Outport
  wires:
  AddSub/1 -> Relational1/1, Gateway Out9/1
  Logical3/1 -> Counter/1
  Counter/1 -> Relational1/2
  IQ valid/1 -> Logical1/2, Logical2/1
  [regRx_NUM_SC] -> AddSub/1
  [regRx_CP_LEN] -> AddSub/2
  Gateway Out9/1 -> Display/1
  Relational1/1 -> Logical1/1
  Logical1/1 -> Sym/1, Logical3/1
  Start/1 -> S-R Latch1/1(S)
  Logical2/1 -> Counter/2
  S-R Latch1/1(Q) -> Logical2/2, Inverter/1
  Rst/1 -> S-R Latch1/2(R)
  Inverter/1 -> Logical3/2

== system wlan_phy_rx_pmd/Sync & Antenna Sel/Pkt Det/Ext Pkt Det/Debouce ==
  D : Inport
  R : Inport  Port=2
  Logical1 : xbsIndex_r4/Logical  logical_function=AND  inputs=4  en=off  latency=0  precision=Full  arith_type=Unsigned  n_bits=8  bin_pt=2  align_bp=on  dbl_ovrd=off  block_type=logical
      settings: xl_area=[1 0 0 1 0 0 0]  block_version=VER_STRING_GOES_HERE
  Register{,1..3} (4 alike) : xbsIndex_r4/Register  init=0  rst=on  en=off  dbl_ovrd=off  block_type=register
  Q : Outport
  wires:
  Logical1/1 -> Q/1
  Register/1 -> Register1/1, Logical1/1
  Register1/1 -> Register2/1, Logical1/2
  Register2/1 -> Register3/1, Logical1/3
  Register3/1 -> Logical1/4
  D/1 -> Register/1
  R/1 -> Register/2, Register1/2, Register2/2, Register3/2

== system wlan_phy_rx_pmd/Sync & Antenna Sel/Pkt Det/Ext Pkt Det/Posedge1 ==
  note: Rising Edge Detection
  D : Inport
  Delay : xbsIndex_r4/Delay  rst=off  infoeditControl=Selection of Reset will increase slice count due to use of real FFs and instead of SRLs  en=off  latency=1  dbl_ovrd=off  reg_retiming=off  block_type=delay
      settings: infoedit=Hardware notes: A delay line is a chain, each link of which is an SRL16 followed by a flip-flop. If register retiming is enabled, the delay line is a chain of flip-flops.  xl_area=[1 1 0 0 0 0 0]  block_version=VER_STRING_GOES_HERE
  Inverter : xbsIndex_r4/Inverter  en=off  latency=0  dbl_ovrd=off  block_type=inv
      settings: xl_area=[0 0 0 0 0 0 0]  block_version=VER_STRING_GOES_HERE
  Logical1 : xbsIndex_r4/Logical  logical_function=AND  inputs=2  en=off  latency=0  precision=Full  arith_type=Unsigned  n_bits=8  bin_pt=2  align_bp=on  dbl_ovrd=off  block_type=logical
      settings: xl_area=[1 0 0 1 0 0 0]  block_version=VER_STRING_GOES_HERE
  Q : Outport
  wires:
  Delay/1 -> Inverter/1
  Inverter/1 -> Logical1/1
  Logical1/1 -> Q/1
  D/1 -> Delay/1, Logical1/2

== system wlan_phy_rx_pmd/Sync & Antenna Sel/Pkt Det/Ext Pkt Det/S-R Latch ==
  R : Inport
  S : Inport  Port=2
  Constant1 : xbsIndex_r4/Constant  const=1  gui_display_data_type=Fixed-point  arith_type=Boolean  n_bits=1  bin_pt=0  preci_type=Single  exp_width=8  frac_width=24  explicit_period=off  period=1  equ=P=C  opselect=C  inp2=PCIN>>17  opr=+  inp1=P  carry=CIN  dbl_ovrd=off  block_type=constant
      settings: block_version=VER_STRING_GOES_HERE
  Register2 : xbsIndex_r4/Register  init=0  rst=on  en=on  dbl_ovrd=off  block_type=register
      settings: xl_area=[1 1 0 0 0 0 0]  block_version=VER_STRING_GOES_HERE
  Q : Outport
  wires:
  Constant1/1 -> Register2/1
  R/1 -> Register2/2
  Register2/1 -> Q/1
  S/1 -> Register2/3

== system wlan_phy_rx_pmd/Sync & Antenna Sel/Pkt Det/RF A Det/Auto-Corr Pkt Det ==
  Reset OFDM : Inport
  Reset DSSS : Inport  Port=2
  rx_I : Inport  Port=3
  rx_Q : Inport  Port=4
  rx_valid : Inport  Port=5
  Auto Corr : Subsystem
  Auto Corr Pkt Det : Scope  NumInputPorts=9
      settings: TimeRange=40000  YMin=-1~-0.5~0~0~0~0~-1~0~0  YMax=1~0.5~1.5~1~0.4~1~1~1~1  SaveName=ScopeData28
  Decision : Subsystem
  Mag : Subsystem
  Register1 : xbsIndex_r4/Register  init=0  rst=off  en=off  dbl_ovrd=off  block_type=register
  Register2 : xbsIndex_r4/Register  init=0  rst=off  en=off  dbl_ovrd=off  block_type=register
  Register3 : xbsIndex_r4/Register  init=0  rst=off  en=off  dbl_ovrd=off  block_type=register
  done{1..9} (9 alike) : xbsIndex_r4/Gateway Out  inherit_from_input=off  hdl_port=off  timing_constraint=None  locs_specified=off  LOCs={}  UseAsDAC=off  DACChannel='1'  block_type=gatewayout
      settings: infoedit="Gateway out block.  Converts Xilinx fixed point inputs into ouputs of type Simulink integer, double, or fixed point.<P><P>Hardware notes:  In hardware these blocks become top level output ports or are discarded, depending on how they are configured."  xl_area=[0 0 0 0 1 0 0]  block_version=VER_STRING_GOES_HERE
  Det OFDM : Outport
  Det DSSS : Outport  Port=2
  wires:
  Decision/1(Det OFDM) -> done6/1, Det OFDM/1
  Mag/1(Mag Sq) -> Decision/5(Mag), done3/1
  Auto Corr/1(Corr_16) -> Decision/3(Corr_16), done5/1
  Register2/1 -> Auto Corr/1(Valid), Mag/1(Valid)
  Register3/1 -> Auto Corr/3(Q), Mag/3(Q), done2/1
  Register1/1 -> Auto Corr/2(I), Mag/2(I), done4/1
  rx_valid/1 -> Register2/1
  rx_I/1 -> Register1/1
  rx_Q/1 -> Register3/1
  Auto Corr/2(Corr_20) -> Decision/4(Corr_20), done7/1
  done4/1 -> Auto Corr Pkt Det/1  "ADCI"
  done2/1 -> Auto Corr Pkt Det/2  "ADCQ"
  done3/1 -> Auto Corr Pkt Det/3  "Mag"
  done5/1 -> Auto Corr Pkt Det/4  "Corr OFDM"
  done7/1 -> Auto Corr Pkt Det/5  "Corr DSSS"
  done6/1 -> Auto Corr Pkt Det/6  "Det OFDM"
  done8/1 -> Auto Corr Pkt Det/7  "Det DSSS"
  done1/1 -> Auto Corr Pkt Det/8  "Det Reset OFDM"
  done9/1 -> Auto Corr Pkt Det/9  "Det Reset DSSS"
  Decision/2(Det DSSS) -> done8/1, Det DSSS/1
  Reset OFDM/1 -> Decision/1(Reset OFDM), done1/1
  Reset DSSS/1 -> Decision/2(Reset DSSS), done9/1

== system wlan_phy_rx_pmd/Sync & Antenna Sel/Pkt Det/RF A Det/RSSI Pkt Det ==
  note: The DSSS and OFDM pipelines operate independently. Both require
      | their pktDet inputs to assert at the beginning of a reception
      | and stay asserted until the pipeline is finished. But each pipeline
      | can reset the other (valid OFDM implies DSSS not valid), so
      | the pkt det state bits must be kept separate here.
  Reset OFDM : Inport
  Reset DSSS : Inport  Port=2
  Avg RSSI : Inport  Port=3
  Detection Decision : Subsystem
  Inverter : xbsIndex_r4/Inverter  en=off  latency=0  dbl_ovrd=off  block_type=inv
  Logical3 : xbsIndex_r4/Logical  logical_function=AND  inputs=2  en=off  latency=0  precision=Full  arith_type=Unsigned  n_bits=8  bin_pt=2  align_bp=on  dbl_ovrd=off  block_type=logical
      settings: xl_area=[1 0 0 1 0 0 0]  block_version=VER_STRING_GOES_HERE
  Posedge1 : Subsystem
  Register6 : xbsIndex_r4/Register  init=0  rst=off  en=off  dbl_ovrd=off  block_type=register
  S-R Latch1 : Subsystem
  S-R Latch2 : Subsystem
  PktDet OFDM : Outport
  PktDet DSSS : Outport  Port=2
  wires:
  Avg RSSI/1 -> Detection Decision/1(Avg RSSI)
  Posedge1/1(Q) -> Logical3/2
  S-R Latch1/1(Q) -> PktDet OFDM/1
  Reset OFDM/1 -> S-R Latch1/2(R)
  Detection Decision/1(Pkt Det) -> Posedge1/1(D)
  S-R Latch2/1(Q) -> PktDet DSSS/1
  Reset DSSS/1 -> S-R Latch2/2(R)
  [MAC_PHY_BLOCK_PKT_DET] -> Inverter/1
  Logical3/1 -> S-R Latch1/1(S), S-R Latch2/1(S)
  Inverter/1 -> Register6/1
  Register6/1 -> Logical3/1
